simulink model "CCMHfaMotor"  (R2022b)
settings: Solver=FixedStepDiscrete  StartTime=0.0  StopTime=10.0  FixedStep=0.01  RelTol=1e-3
model workspace (MAT data in the model file):
  HfaWakeUpFlag : Simulink.Signal (value not decoded)
  NvMBlockHfaMtrCalData : Simulink.Signal (value not decoded)
  NvMBlockHfaMtrCalDataInit : Simulink.Parameter (value not decoded)
symbols that are objects in the model workspace (value not decoded): NvMBlockHfaMtrCalDataInit
undefined symbols (not defined in the file or in its folder): ComChlType, DioChannelType, HFAChlType, LinFrameType, NetworkChlType, NvMBlockId, P_DioLevelType, P_LinIfSchHandleType, P_Std_ReturnType, PowerChlType
(part 3 of 4)

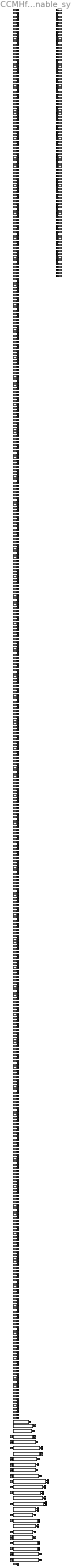
== system CCMHfaMotor/HfaMotor_Runnable_sys ==
  AcRfrPrgSafety_AcRfrPrgSafety_read : Inport
  ActRfrIdeGroup_ActRfrIdeGroup_read : Inport  Port=2
  ActRfrIdeItem_ActRfrIdeItem_read : Inport  Port=3
  ActRfrIdePN_ActRfrIdePN_read : Inport  Port=4
  ActRfrIdeSupplier_ActRfrIdeSupplier_read : Inport  Port=5
  ActRfrIdeVers_ActRfrIdeVers_read : Inport  Port=6
  ActRfrPrgDiret_ActRfrPrgDiret_read : Inport  Port=7
  ActRfrPrgGroup_ActRfrPrgGroup_read : Inport  Port=8
  ActRfrPrgItem_ActRfrPrgItem_read : Inport  Port=9
  ActRfrPrgPrgGroup_ActRfrPrgPrgGroup_read : Inport  Port=10
  ActRfrPrgPrgItem_ActRfrPrgPrgItem_read : Inport  Port=11
  ActRfrPrgRef_ActRfrPrgRef_read : Inport  Port=12
  ActRfrPrgSafetyEnable_ActRfrPrgSafetyEnable_read : Inport  Port=13
  ActRfrStaCPos_ActRfrStaCPos_read : Inport  Port=14
  ActRfrStaEmgyRun_ActRfrStaEmgyRun_read : Inport  Port=15
  ActRfrStaErr_ActRfrStaErr_read : Inport  Port=16
  ActRfrStaGroup_ActRfrStaGroup_read : Inport  Port=17
  ActRfrStaItem_ActRfrStaItem_read : Inport  Port=18
  ActRfrStaMod_ActRfrStaMod_read : Inport  Port=19
  ActRfrStaReset_ActRfrStaReset_read : Inport  Port=20
  ActRfrStaStd_ActRfrStaStd_read : Inport  Port=21
  BatSysBtrMSts_BatSysBtrMSts_read : Inport  Port=22
  HfaCcmSlaveRespB0Lin4_HfaCcmSlaveRespB0Lin4_read : Inport  Port=23
  HfaCcmSlaveRespB1Lin4_HfaCcmSlaveRespB1Lin4_read : Inport  Port=24
  HfaCcmSlaveRespB2Lin4_HfaCcmSlaveRespB2Lin4_read : Inport  Port=25
  HfaCcmSlaveRespB3Lin4_HfaCcmSlaveRespB3Lin4_read : Inport  Port=26
  HfaCcmSlaveRespB4Lin4_HfaCcmSlaveRespB4Lin4_read : Inport  Port=27
  HfaCcmSlaveRespB5Lin4_HfaCcmSlaveRespB5Lin4_read : Inport  Port=28
  HfaCcmSlaveRespB6Lin4_HfaCcmSlaveRespB6Lin4_read : Inport  Port=29
  HfaCcmSlaveRespB7Lin4_HfaCcmSlaveRespB7Lin4_read : Inport  Port=30
  HmiElecAirSwngReq_HmiElecAirSwngReq_read : Inport  Port=31
  HvacCfgForClimaCtrl_HvacCfgForHvacFrontBlowerType_read : Inport  Port=32
  HvacCfgForClimaCtrl_HvacCfgForHvacMiscExistSts_read : Inport  Port=33
  HvacCfgForClimaCtrl_HvacCfgForHvacMotorExistSts_read : Inport  Port=34
  HvacCfgForClimaCtrl_HvacCfgForHvacSensorExistSts_read : Inport  Port=35
  SbcPwrOn_SbcPwrOn_read : Inport  Port=36
  SbcWakeupSta_SbcWakeupSta_read : Inport  Port=37
  SysServCarCfgSts_SysServCarID_read : Inport  Port=38
  SysServCarCfgSts_SysServCCPAQSSts_read : Inport  Port=39
  SysServCarCfgSts_SysServCCPHEXSensorSts_read : Inport  Port=40
  SysServCarCfgSts_SysServCCPLin2AFUSts_read : Inport  Port=41
  SysServCarCfgSts_SysServCCPLin2AIUSts_read : Inport  Port=42
  SysServCarCfgSts_SysServCCPLin2CDSSts_read : Inport  Port=43
  SysServCarCfgSts_SysServCCPLin2PMSISts_read : Inport  Port=44
  SysServCarCfgSts_SysServCCPLin3VGASts_read : Inport  Port=45
  SysServCarCfgSts_SysServDUAirVentSnrSts_read : Inport  Port=46
  SysServCarCfgSts_SysServDUBlowerPwmSignSts_read : Inport  Port=47
  SysServCarCfgSts_SysServDUBlowerPwmSts_read : Inport  Port=48
  SysServCarCfgSts_SysServDUDCDefrostSts_read : Inport  Port=49
  SysServCarCfgSts_SysServDUDCModeSts_read : Inport  Port=50
  SysServCarCfgSts_SysServDUDCRecSts_read : Inport  Port=51
  SysServCarCfgSts_SysServDUDCSModeSts_read : Inport  Port=52
  SysServCarCfgSts_SysServDUDCSTLSts_read : Inport  Port=53
  SysServCarCfgSts_SysServDUDCTLSts_read : Inport  Port=54
  SysServCarCfgSts_SysServDUDCTRSts_read : Inport  Port=55
  SysServCarCfgSts_SysServDUFLFootSts_read : Inport  Port=56
  SysServCarCfgSts_SysServDUFLVentSts_read : Inport  Port=57
  SysServCarCfgSts_SysServDUFRFootSts_read : Inport  Port=58
  SysServCarCfgSts_SysServDUFRVentSts_read : Inport  Port=59
  SysServCarCfgSts_SysServDUIncarMotorSts_read : Inport  Port=60
  SysServCarCfgSts_SysServDULin1HBMFSts_read : Inport  Port=61
  SysServCarCfgSts_SysServDULin4HFAFSts_read : Inport  Port=62
  SysServCarCfgSts_SysServDUSFLFootSts_read : Inport  Port=63
  SysServCarCfgSts_SysServDUSFLVentSts_read : Inport  Port=64
  SysServCarCfgSts_SysServDUSFRFootSts_read : Inport  Port=65
  SysServCarCfgSts_SysServDUSFRVentSts_read : Inport  Port=66
  SysServCarCfgSts_SysServDUSnsrForFCmptmtTSts_read : Inport  Port=67
  SysServCarCfgSts_SysServDUSnsrForRair_read : Inport  Port=68
  SysServCarCfgSts_SysServDUSnsrForRCmptmtTSts_read : Inport  Port=69
  BatLoadBtrMSts_BatLoadBtrMSts_read : Inport  Port=70
  HfaMotSetPowerOnInit_PowerOnInit_read : Inport  Port=71
  VehModMngtGlbSafe1_VehModMngtGlbSafe1_read : Inport  Port=72
  HfaActr01ActPson_HfaActr01ActPson_read : Inport  Port=73
  HfaActr01ActPson_HfaActr01ActPson_IsUpdated_read : Inport  Port=74
  HfaActr01BlckInd_HfaActr01BlckInd_read : Inport  Port=75
  HfaActr01BlckSts_HfaActr01BlckSts_read : Inport  Port=76
  HfaActr01BusAdr_HfaActr01BusAdr_read : Inport  Port=77
  HfaActr01CoilSts_HfaActr01CoilSts_read : Inport  Port=78
  HfaActr01DrvDir_HfaActr01DrvDir_read : Inport  Port=79
  HfaActr01ElecErr_HfaActr01ElecErr_read : Inport  Port=80
  HfaActr01Mode_HfaActr01Mode_read : Inport  Port=81
  HfaActr01MovDir_HfaActr01MovDir_read : Inport  Port=82
  HfaActr01OvrTempErr_HfaActr01OvrTempErr_read : Inport  Port=83
  HfaActr01PsonSts_HfaActr01PsonSts_read : Inport  Port=84
  HfaActr01ResetSts_HfaActr01ResetSts_read : Inport  Port=85
  HfaActr01SpclFct_HfaActr01SpclFct_read : Inport  Port=86
  HfaActr01SpdSts_HfaActr01SpdSts_read : Inport  Port=87
  HfaActr01TrqSts_HfaActr01TrqSts_read : Inport  Port=88
  HfaActr01UrgcExist_HfaActr01UrgcExist_read : Inport  Port=89
  HfaActr01UrgcPson_HfaActr01UrgcPson_read : Inport  Port=90
  HfaActr01UrgcSts_HfaActr01UrgcSts_read : Inport  Port=91
  HfaActr01VoltErr_HfaActr01VoltErr_read : Inport  Port=92
  HfaActr02ActPson_HfaActr02ActPson_read : Inport  Port=93
  HfaActr02ActPson_HfaActr02ActPson_IsUpdated_read : Inport  Port=94
  HfaActr02BlckInd_HfaActr02BlckInd_read : Inport  Port=95
  HfaActr02BlckSts_HfaActr02BlckSts_read : Inport  Port=96
  HfaActr02BusAdr_HfaActr02BusAdr_read : Inport  Port=97
  HfaActr02CoilSts_HfaActr02CoilSts_read : Inport  Port=98
  HfaActr02DrvDir_HfaActr02DrvDir_read : Inport  Port=99
  HfaActr02ElecErr_HfaActr02ElecErr_read : Inport  Port=100
  HfaActr02Mode_HfaActr02Mode_read : Inport  Port=101
  HfaActr02MovDir_HfaActr02MovDir_read : Inport  Port=102
  HfaActr02OvrTempErr_HfaActr02OvrTempErr_read : Inport  Port=103
  HfaActr02PsonSts_HfaActr02PsonSts_read : Inport  Port=104
  HfaActr02ResetSts_HfaActr02ResetSts_read : Inport  Port=105
  HfaActr02SpclFct_HfaActr02SpclFct_read : Inport  Port=106
  HfaActr02SpdSts_HfaActr02SpdSts_read : Inport  Port=107
  HfaActr02TrqSts_HfaActr02TrqSts_read : Inport  Port=108
  HfaActr02UrgcExist_HfaActr02UrgcExist_read : Inport  Port=109
  HfaActr02UrgcPson_HfaActr02UrgcPson_read : Inport  Port=110
  HfaActr02UrgcSts_HfaActr02UrgcSts_read : Inport  Port=111
  HfaActr02VoltErr_HfaActr02VoltErr_read : Inport  Port=112
  HfaActr03ActPson_HfaActr03ActPson_read : Inport  Port=113
  HfaActr03ActPson_HfaActr03ActPson_IsUpdated_read : Inport  Port=114
  HfaActr03BlckInd_HfaActr03BlckInd_read : Inport  Port=115
  HfaActr03BlckSts_HfaActr03BlckSts_read : Inport  Port=116
  HfaActr03BusAdr_HfaActr03BusAdr_read : Inport  Port=117
  HfaActr03CoilSts_HfaActr03CoilSts_read : Inport  Port=118
  HfaActr03DrvDir_HfaActr03DrvDir_read : Inport  Port=119
  HfaActr03ElecErr_HfaActr03ElecErr_read : Inport  Port=120
  HfaActr03Mode_HfaActr03Mode_read : Inport  Port=121
  HfaActr03MovDir_HfaActr03MovDir_read : Inport  Port=122
  HfaActr03OvrTempErr_HfaActr03OvrTempErr_read : Inport  Port=123
  HfaActr03PsonSts_HfaActr03PsonSts_read : Inport  Port=124
  HfaActr03ResetSts_HfaActr03ResetSts_read : Inport  Port=125
  HfaActr03SpclFct_HfaActr03SpclFct_read : Inport  Port=126
  HfaActr03SpdSts_HfaActr03SpdSts_read : Inport  Port=127
  HfaActr03TrqSts_HfaActr03TrqSts_read : Inport  Port=128
  HfaActr03UrgcExist_HfaActr03UrgcExist_read : Inport  Port=129
  HfaActr03UrgcPson_HfaActr03UrgcPson_read : Inport  Port=130
  HfaActr03UrgcSts_HfaActr03UrgcSts_read : Inport  Port=131
  HfaActr03VoltErr_HfaActr03VoltErr_read : Inport  Port=132
  HfaActr04ActPson_HfaActr04ActPson_read : Inport  Port=133
  HfaActr04ActPson_HfaActr04ActPson_IsUpdated_read : Inport  Port=134
  HfaActr04BlckInd_HfaActr04BlckInd_read : Inport  Port=135
  HfaActr04BlckSts_HfaActr04BlckSts_read : Inport  Port=136
  HfaActr04BusAdr_HfaActr04BusAdr_read : Inport  Port=137
  HfaActr04CoilSts_HfaActr04CoilSts_read : Inport  Port=138
  HfaActr04DrvDir_HfaActr04DrvDir_read : Inport  Port=139
  HfaActr04ElecErr_HfaActr04ElecErr_read : Inport  Port=140
  HfaActr04Mode_HfaActr04Mode_read : Inport  Port=141
  HfaActr04MovDir_HfaActr04MovDir_read : Inport  Port=142
  HfaActr04OvrTempErr_HfaActr04OvrTempErr_read : Inport  Port=143
  HfaActr04PsonSts_HfaActr04PsonSts_read : Inport  Port=144
  HfaActr04ResetSts_HfaActr04ResetSts_read : Inport  Port=145
  HfaActr04SpclFct_HfaActr04SpclFct_read : Inport  Port=146
  HfaActr04SpdSts_HfaActr04SpdSts_read : Inport  Port=147
  HfaActr04TrqSts_HfaActr04TrqSts_read : Inport  Port=148
  HfaActr04UrgcExist_HfaActr04UrgcExist_read : Inport  Port=149
  HfaActr04UrgcPson_HfaActr04UrgcPson_read : Inport  Port=150
  HfaActr04UrgcSts_HfaActr04UrgcSts_read : Inport  Port=151
  HfaActr04VoltErr_HfaActr04VoltErr_read : Inport  Port=152
  HfaActr05ActPson_HfaActr05ActPson_read : Inport  Port=153
  HfaActr05ActPson_HfaActr05ActPson_IsUpdated_read : Inport  Port=154
  HfaActr05BlckInd_HfaActr05BlckInd_read : Inport  Port=155
  HfaActr05BlckSts_HfaActr05BlckSts_read : Inport  Port=156
  HfaActr05BusAdr_HfaActr05BusAdr_read : Inport  Port=157
  HfaActr05CoilSts_HfaActr05CoilSts_read : Inport  Port=158
  HfaActr05DrvDir_HfaActr05DrvDir_read : Inport  Port=159
  HfaActr05ElecErr_HfaActr05ElecErr_read : Inport  Port=160
  HfaActr05Mode_HfaActr05Mode_read : Inport  Port=161
  HfaActr05MovDir_HfaActr05MovDir_read : Inport  Port=162
  HfaActr05OvrTempErr_HfaActr05OvrTempErr_read : Inport  Port=163
  HfaActr05PsonSts_HfaActr05PsonSts_read : Inport  Port=164
  HfaActr05ResetSts_HfaActr05ResetSts_read : Inport  Port=165
  HfaActr05SpclFct_HfaActr05SpclFct_read : Inport  Port=166
  HfaActr05SpdSts_HfaActr05SpdSts_read : Inport  Port=167
  HfaActr05TrqSts_HfaActr05TrqSts_read : Inport  Port=168
  HfaActr05UrgcExist_HfaActr05UrgcExist_read : Inport  Port=169
  HfaActr05UrgcPson_HfaActr05UrgcPson_read : Inport  Port=170
  HfaActr05UrgcSts_HfaActr05UrgcSts_read : Inport  Port=171
  HfaActr05VoltErr_HfaActr05VoltErr_read : Inport  Port=172
  HfaActr06ActPson_HfaActr06ActPson_read : Inport  Port=173
  HfaActr06ActPson_HfaActr06ActPson_IsUpdated_read : Inport  Port=174
  HfaActr06BlckInd_HfaActr06BlckInd_read : Inport  Port=175
  HfaActr06BlckSts_HfaActr06BlckSts_read : Inport  Port=176
  HfaActr06BusAdr_HfaActr06BusAdr_read : Inport  Port=177
  HfaActr06CoilSts_HfaActr06CoilSts_read : Inport  Port=178
  HfaActr06DrvDir_HfaActr06DrvDir_read : Inport  Port=179
  HfaActr06ElecErr_HfaActr06ElecErr_read : Inport  Port=180
  HfaActr06Mode_HfaActr06Mode_read : Inport  Port=181
  HfaActr06MovDir_HfaActr06MovDir_read : Inport  Port=182
  HfaActr06OvrTempErr_HfaActr06OvrTempErr_read : Inport  Port=183
  HfaActr06PsonSts_HfaActr06PsonSts_read : Inport  Port=184
  HfaActr06ResetSts_HfaActr06ResetSts_read : Inport  Port=185
  HfaActr06SpclFct_HfaActr06SpclFct_read : Inport  Port=186
  HfaActr06SpdSts_HfaActr06SpdSts_read : Inport  Port=187
  HfaActr06TrqSts_HfaActr06TrqSts_read : Inport  Port=188
  HfaActr06UrgcExist_HfaActr06UrgcExist_read : Inport  Port=189
  HfaActr06UrgcPson_HfaActr06UrgcPson_read : Inport  Port=190
  HfaActr06UrgcSts_HfaActr06UrgcSts_read : Inport  Port=191
  HfaActr06VoltErr_HfaActr06VoltErr_read : Inport  Port=192
  HfaActr07ActPson_HfaActr07ActPson_read : Inport  Port=193
  HfaActr07ActPson_HfaActr07ActPson_IsUpdated_read : Inport  Port=194
  HfaActr07BlckInd_HfaActr07BlckInd_read : Inport  Port=195
  HfaActr07BlckSts_HfaActr07BlckSts_read : Inport  Port=196
  HfaActr07BusAdr_HfaActr07BusAdr_read : Inport  Port=197
  HfaActr07CoilSts_HfaActr07CoilSts_read : Inport  Port=198
  HfaActr07DrvDir_HfaActr07DrvDir_read : Inport  Port=199
  HfaActr07ElecErr_HfaActr07ElecErr_read : Inport  Port=200
  HfaActr07Mode_HfaActr07Mode_read : Inport  Port=201
  HfaActr07MovDir_HfaActr07MovDir_read : Inport  Port=202
  HfaActr07OvrTempErr_HfaActr07OvrTempErr_read : Inport  Port=203
  HfaActr07PsonSts_HfaActr07PsonSts_read : Inport  Port=204
  HfaActr07ResetSts_HfaActr07ResetSts_read : Inport  Port=205
  HfaActr07SpclFct_HfaActr07SpclFct_read : Inport  Port=206
  HfaActr07SpdSts_HfaActr07SpdSts_read : Inport  Port=207
  HfaActr07TrqSts_HfaActr07TrqSts_read : Inport  Port=208
  HfaActr07UrgcExist_HfaActr07UrgcExist_read : Inport  Port=209
  HfaActr07UrgcPson_HfaActr07UrgcPson_read : Inport  Port=210
  HfaActr07UrgcSts_HfaActr07UrgcSts_read : Inport  Port=211
  HfaActr07VoltErr_HfaActr07VoltErr_read : Inport  Port=212
  HfaActr08ActPson_HfaActr08ActPson_read : Inport  Port=213
  HfaActr08ActPson_HfaActr08ActPson_IsUpdated_read : Inport  Port=214
  HfaActr08BlckInd_HfaActr08BlckInd_read : Inport  Port=215
  HfaActr08BlckSts_HfaActr08BlckSts_read : Inport  Port=216
  HfaActr08BusAdr_HfaActr08BusAdr_read : Inport  Port=217
  HfaActr08CoilSts_HfaActr08CoilSts_read : Inport  Port=218
  HfaActr08DrvDir_HfaActr08DrvDir_read : Inport  Port=219
  HfaActr08ElecErr_HfaActr08ElecErr_read : Inport  Port=220
  HfaActr08Mode_HfaActr08Mode_read : Inport  Port=221
  HfaActr08MovDir_HfaActr08MovDir_read : Inport  Port=222
  HfaActr08OvrTempErr_HfaActr08OvrTempErr_read : Inport  Port=223
  HfaActr08PsonSts_HfaActr08PsonSts_read : Inport  Port=224
  HfaActr08ResetSts_HfaActr08ResetSts_read : Inport  Port=225
  HfaActr08SpclFct_HfaActr08SpclFct_read : Inport  Port=226
  HfaActr08SpdSts_HfaActr08SpdSts_read : Inport  Port=227
  HfaActr08TrqSts_HfaActr08TrqSts_read : Inport  Port=228
  HfaActr08UrgcExist_HfaActr08UrgcExist_read : Inport  Port=229
  HfaActr08UrgcPson_HfaActr08UrgcPson_read : Inport  Port=230
  HfaActr08UrgcSts_HfaActr08UrgcSts_read : Inport  Port=231
  HfaActr08VoltErr_HfaActr08VoltErr_read : Inport  Port=232
  HfaActr09ActPson_HfaActr09ActPson_read : Inport  Port=233
  HfaActr09ActPson_HfaActr09ActPson_IsUpdated_read : Inport  Port=234
  HfaActr09BlckInd_HfaActr09BlckInd_read : Inport  Port=235
  HfaActr09BlckSts_HfaActr09BlckSts_read : Inport  Port=236
  HfaActr09BusAdr_HfaActr09BusAdr_read : Inport  Port=237
  HfaActr09CoilSts_HfaActr09CoilSts_read : Inport  Port=238
  HfaActr09DrvDir_HfaActr09DrvDir_read : Inport  Port=239
  HfaActr09ElecErr_HfaActr09ElecErr_read : Inport  Port=240
  HfaActr09Mode_HfaActr09Mode_read : Inport  Port=241
  HfaActr09MovDir_HfaActr09MovDir_read : Inport  Port=242
  HfaActr09OvrTempErr_HfaActr09OvrTempErr_read : Inport  Port=243
  HfaActr09PsonSts_HfaActr09PsonSts_read : Inport  Port=244
  HfaActr09ResetSts_HfaActr09ResetSts_read : Inport  Port=245
  HfaActr09SpclFct_HfaActr09SpclFct_read : Inport  Port=246
  HfaActr09SpdSts_HfaActr09SpdSts_read : Inport  Port=247
  HfaActr09TrqSts_HfaActr09TrqSts_read : Inport  Port=248
  HfaActr09UrgcExist_HfaActr09UrgcExist_read : Inport  Port=249
  HfaActr09UrgcPson_HfaActr09UrgcPson_read : Inport  Port=250
  HfaActr09UrgcSts_HfaActr09UrgcSts_read : Inport  Port=251
  HfaActr09VoltErr_HfaActr09VoltErr_read : Inport  Port=252
  HfaActr10ActPson_HfaActr10ActPson_read : Inport  Port=253
  HfaActr10ActPson_HfaActr10ActPson_IsUpdated_read : Inport  Port=254
  HfaActr10BlckInd_HfaActr10BlckInd_read : Inport  Port=255
  HfaActr10BlckSts_HfaActr10BlckSts_read : Inport  Port=256
  HfaActr10BusAdr_HfaActr10BusAdr_read : Inport  Port=257
  HfaActr10CoilSts_HfaActr10CoilSts_read : Inport  Port=258
  HfaActr10DrvDir_HfaActr10DrvDir_read : Inport  Port=259
  HfaActr10ElecErr_HfaActr10ElecErr_read : Inport  Port=260
  HfaActr10Mode_HfaActr10Mode_read : Inport  Port=261
  HfaActr10MovDir_HfaActr10MovDir_read : Inport  Port=262
  HfaActr10OvrTempErr_HfaActr10OvrTempErr_read : Inport  Port=263
  HfaActr10PsonSts_HfaActr10PsonSts_read : Inport  Port=264
  HfaActr10ResetSts_HfaActr10ResetSts_read : Inport  Port=265
  HfaActr10SpclFct_HfaActr10SpclFct_read : Inport  Port=266
  HfaActr10SpdSts_HfaActr10SpdSts_read : Inport  Port=267
  HfaActr10TrqSts_HfaActr10TrqSts_read : Inport  Port=268
  HfaActr10UrgcExist_HfaActr10UrgcExist_read : Inport  Port=269
  HfaActr10UrgcPson_HfaActr10UrgcPson_read : Inport  Port=270
  HfaActr10UrgcSts_HfaActr10UrgcSts_read : Inport  Port=271
  HfaActr10VoltErr_HfaActr10VoltErr_read : Inport  Port=272
  HfaActr11ActPson_HfaActr11ActPson_read : Inport  Port=273
  HfaActr11ActPson_HfaActr11ActPson_IsUpdated_read : Inport  Port=274
  HfaActr11BlckInd_HfaActr11BlckInd_read : Inport  Port=275
  HfaActr11BlckSts_HfaActr11BlckSts_read : Inport  Port=276
  HfaActr11BusAdr_HfaActr11BusAdr_read : Inport  Port=277
  HfaActr11CoilSts_HfaActr11CoilSts_read : Inport  Port=278
  HfaActr11DrvDir_HfaActr11DrvDir_read : Inport  Port=279
  HfaActr11ElecErr_HfaActr11ElecErr_read : Inport  Port=280
  HfaActr11Mode_HfaActr11Mode_read : Inport  Port=281
  HfaActr11MovDir_HfaActr11MovDir_read : Inport  Port=282
  HfaActr11OvrTempErr_HfaActr11OvrTempErr_read : Inport  Port=283
  HfaActr11PsonSts_HfaActr11PsonSts_read : Inport  Port=284
  HfaActr11ResetSts_HfaActr11ResetSts_read : Inport  Port=285
  HfaActr11SpclFct_HfaActr11SpclFct_read : Inport  Port=286
  HfaActr11SpdSts_HfaActr11SpdSts_read : Inport  Port=287
  HfaActr11TrqSts_HfaActr11TrqSts_read : Inport  Port=288
  HfaActr11UrgcExist_HfaActr11UrgcExist_read : Inport  Port=289
  HfaActr11UrgcPson_HfaActr11UrgcPson_read : Inport  Port=290
  HfaActr11UrgcSts_HfaActr11UrgcSts_read : Inport  Port=291
  HfaActr11VoltErr_HfaActr11VoltErr_read : Inport  Port=292
  HfaActr12ActPson_HfaActr12ActPson_read : Inport  Port=293
  HfaActr12ActPson_HfaActr12ActPson_IsUpdated_read : Inport  Port=294
  HfaActr12BlckInd_HfaActr12BlckInd_read : Inport  Port=295
  HfaActr12BlckSts_HfaActr12BlckSts_read : Inport  Port=296
  HfaActr12BusAdr_HfaActr12BusAdr_read : Inport  Port=297
  HfaActr12CoilSts_HfaActr12CoilSts_read : Inport  Port=298
  HfaActr12DrvDir_HfaActr12DrvDir_read : Inport  Port=299
  HfaActr12ElecErr_HfaActr12ElecErr_read : Inport  Port=300
  HfaActr12Mode_HfaActr12Mode_read : Inport  Port=301
  HfaActr12MovDir_HfaActr12MovDir_read : Inport  Port=302
  HfaActr12OvrTempErr_HfaActr12OvrTempErr_read : Inport  Port=303
  HfaActr12PsonSts_HfaActr12PsonSts_read : Inport  Port=304
  HfaActr12ResetSts_HfaActr12ResetSts_read : Inport  Port=305
  HfaActr12SpclFct_HfaActr12SpclFct_read : Inport  Port=306
  HfaActr12SpdSts_HfaActr12SpdSts_read : Inport  Port=307
  HfaActr12TrqSts_HfaActr12TrqSts_read : Inport  Port=308
  HfaActr12UrgcExist_HfaActr12UrgcExist_read : Inport  Port=309
  HfaActr12UrgcPson_HfaActr12UrgcPson_read : Inport  Port=310
  HfaActr12UrgcSts_HfaActr12UrgcSts_read : Inport  Port=311
  HfaActr12VoltErr_HfaActr12VoltErr_read : Inport  Port=312
  HfaActr13ActPson_HfaActr13ActPson_read : Inport  Port=313
  HfaActr13ActPson_HfaActr13ActPson_IsUpdated_read : Inport  Port=314
  HfaActr13BlckInd_HfaActr13BlckInd_read : Inport  Port=315
  HfaActr13BlckSts_HfaActr13BlckSts_read : Inport  Port=316
  HfaActr13BusAdr_HfaActr13BusAdr_read : Inport  Port=317
  HfaActr13CoilSts_HfaActr13CoilSts_read : Inport  Port=318
  HfaActr13DrvDir_HfaActr13DrvDir_read : Inport  Port=319
  HfaActr13ElecErr_HfaActr13ElecErr_read : Inport  Port=320
  HfaActr13Mode_HfaActr13Mode_read : Inport  Port=321
  HfaActr13MovDir_HfaActr13MovDir_read : Inport  Port=322
  HfaActr13OvrTempErr_HfaActr13OvrTempErr_read : Inport  Port=323
  HfaActr13PsonSts_HfaActr13PsonSts_read : Inport  Port=324
  HfaActr13ResetSts_HfaActr13ResetSts_read : Inport  Port=325
  HfaActr13SpclFct_HfaActr13SpclFct_read : Inport  Port=326
  HfaActr13SpdSts_HfaActr13SpdSts_read : Inport  Port=327
  HfaActr13TrqSts_HfaActr13TrqSts_read : Inport  Port=328
  HfaActr13UrgcExist_HfaActr13UrgcExist_read : Inport  Port=329
  HfaActr13UrgcPson_HfaActr13UrgcPson_read : Inport  Port=330
  HfaActr13UrgcSts_HfaActr13UrgcSts_read : Inport  Port=331
  HfaActr13VoltErr_HfaActr13VoltErr_read : Inport  Port=332
  HfaActr14ActPson_HfaActr14ActPson_read : Inport  Port=333
  HfaActr14ActPson_HfaActr14ActPson_IsUpdated_read : Inport  Port=334
  HfaActr14BlckInd_HfaActr14BlckInd_read : Inport  Port=335
  HfaActr14BlckSts_HfaActr14BlckSts_read : Inport  Port=336
  HfaActr14BusAdr_HfaActr14BusAdr_read : Inport  Port=337
  HfaActr14CoilSts_HfaActr14CoilSts_read : Inport  Port=338
  HfaActr14DrvDir_HfaActr14DrvDir_read : Inport  Port=339
  HfaActr14ElecErr_HfaActr14ElecErr_read : Inport  Port=340
  HfaActr14Mode_HfaActr14Mode_read : Inport  Port=341
  HfaActr14MovDir_HfaActr14MovDir_read : Inport  Port=342
  HfaActr14OvrTempErr_HfaActr14OvrTempErr_read : Inport  Port=343
  HfaActr14PsonSts_HfaActr14PsonSts_read : Inport  Port=344
  HfaActr14ResetSts_HfaActr14ResetSts_read : Inport  Port=345
  HfaActr14SpclFct_HfaActr14SpclFct_read : Inport  Port=346
  HfaActr14SpdSts_HfaActr14SpdSts_read : Inport  Port=347
  HfaActr14TrqSts_HfaActr14TrqSts_read : Inport  Port=348
  HfaActr14UrgcExist_HfaActr14UrgcExist_read : Inport  Port=349
  HfaActr14UrgcPson_HfaActr14UrgcPson_read : Inport  Port=350
  HfaActr14UrgcSts_HfaActr14UrgcSts_read : Inport  Port=351
  HfaActr14VoltErr_HfaActr14VoltErr_read : Inport  Port=352
  HfaActr15ActPson_HfaActr15ActPson_read : Inport  Port=353
  HfaActr15ActPson_HfaActr15ActPson_IsUpdated_read : Inport  Port=354
  HfaActr15BlckInd_HfaActr15BlckInd_read : Inport  Port=355
  HfaActr15BlckSts_HfaActr15BlckSts_read : Inport  Port=356
  HfaActr15BusAdr_HfaActr15BusAdr_read : Inport  Port=357
  HfaActr15CoilSts_HfaActr15CoilSts_read : Inport  Port=358
  HfaActr15DrvDir_HfaActr15DrvDir_read : Inport  Port=359
  HfaActr15ElecErr_HfaActr15ElecErr_read : Inport  Port=360
  HfaActr15Mode_HfaActr15Mode_read : Inport  Port=361
  HfaActr15MovDir_HfaActr15MovDir_read : Inport  Port=362
  HfaActr15OvrTempErr_HfaActr15OvrTempErr_read : Inport  Port=363
  HfaActr15PsonSts_HfaActr15PsonSts_read : Inport  Port=364
  HfaActr15ResetSts_HfaActr15ResetSts_read : Inport  Port=365
  HfaActr15SpclFct_HfaActr15SpclFct_read : Inport  Port=366
  HfaActr15SpdSts_HfaActr15SpdSts_read : Inport  Port=367
  HfaActr15TrqSts_HfaActr15TrqSts_read : Inport  Port=368
  HfaActr15UrgcExist_HfaActr15UrgcExist_read : Inport  Port=369
  HfaActr15UrgcPson_HfaActr15UrgcPson_read : Inport  Port=370
  HfaActr15UrgcSts_HfaActr15UrgcSts_read : Inport  Port=371
  HfaActr15VoltErr_HfaActr15VoltErr_read : Inport  Port=372
  HvacCfgForClimaCtrl_HvacCfgForAQSTypes_read : Inport  Port=373
  HvacCfgForClimaCtrl_HvacCfgForHvacLowSideRETXVESts_read : Inport  Port=374
  HvacCfgForClimaCtrl_HvacCfgForHvacReBlowerType_read : Inport  Port=375
  HvacCfgForClimaCtrl_HvacCfgForHvacReVentPstn_read : Inport  Port=376
  HvacCfgForClimaCtrl_HvacCfgForHvacSnsrRCmptmtESts_read : Inport  Port=377
  HvacCfgForClimaCtrl_HvacCfgForHvacSnsrReHexESts_read : Inport  Port=378
  Lin1ActvReady_Lin1ActvReady_read : Inport  Port=379
  Lin2ActvReady_Lin2ActvReady_read : Inport  Port=380
  ReHvacActrActPson_ReHvacActrActPson_read : Inport  Port=381
  ReHvacActrActPson_ReHvacActrActPson_IsUpdated_read : Inport  Port=382
  ReHvacActrBlckInd_ReHvacActrBlckInd_read : Inport  Port=383
  ReHvacActrBlckSts_ReHvacActrBlckSts_read : Inport  Port=384
  ReHvacActrBusAdr_ReHvacActrBusAdr_read : Inport  Port=385
  ReHvacActrCoilSts_ReHvacActrCoilSts_read : Inport  Port=386
  ReHvacActrDrvDir_ReHvacActrDrvDir_read : Inport  Port=387
  ReHvacActrElecErr_ReHvacActrElecErr_read : Inport  Port=388
  ReHvacActrMode_ReHvacActrMode_read : Inport  Port=389
  ReHvacActrMovDir_ReHvacActrMovDir_read : Inport  Port=390
  ReHvacActrOvrTempErr_ReHvacActrOvrTempErr_read : Inport  Port=391
  ReHvacActrPsonSts_ReHvacActrPsonSts_read : Inport  Port=392
  ReHvacActrResetSts_ReHvacActrResetSts_read : Inport  Port=393
  ReHvacActrSpclFct_ReHvacActrSpclFct_read : Inport  Port=394
  ReHvacActrSpdSts_ReHvacActrSpdSts_read : Inport  Port=395
  ReHvacActrTrqSts_ReHvacActrTrqSts_read : Inport  Port=396
  ReHvacActrUrgcExist_ReHvacActrUrgcExist_read : Inport  Port=397
  ReHvacActrUrgcPson_ReHvacActrUrgcPson_read : Inport  Port=398
  ReHvacActrUrgcSts_ReHvacActrUrgcSts_read : Inport  Port=399
  ReHvacActrVoltErr_ReHvacActrVoltErr_read : Inport  Port=400
  SysServCarCfgSts_SysServCCPBGMNum_read : Inport  Port=401
  SysServCarCfgSts_SysServCCPLin2AQSSts_read : Inport  Port=402
  SysServCarCfgSts_SysServDUBlowerPwmSignTypeSts_read : Inport  Port=403
  SysServCarCfgSts_SysServDUDCFLMdeSts_read : Inport  Port=404
  SysServCarCfgSts_SysServDULin4HFAFStsH_read : Inport  Port=405
  SysServCarCfgSts_SysServDUReBlowerPwmSignSts_read : Inport  Port=406
  SysServCarCfgSts_SysServDUReBlowerPwmSts_read : Inport  Port=407
  SysServCarCfgSts_SysServFloatingMotorSts_read : Inport  Port=408
  SysServCarCfgSts_SysServFrntHEXSnsrPosn_read : Inport  Port=409
  SysServCarCfgSts_SysServLowSideRETXVSts_read : Inport  Port=410
  SysServCarCfgSts_SysServReHEXSnsrPosn_read : Inport  Port=411
  SysServCarCfgSts_SysServRHFASts_read : Inport  Port=412
  SysServCarCfgSts_SysServSnsrForFCmptmtTType_read : Inport  Port=413
  WtrActrLeActPson_WtrActrLeActPson_read : Inport  Port=414
  WtrActrLeBlckInd_WtrActrLeBlckInd_read : Inport  Port=415
  WtrActrLeBlckSts_WtrActrLeBlckSts_read : Inport  Port=416
  WtrActrLeBusAdr_WtrActrLeBusAdr_read : Inport  Port=417
  WtrActrLeCoilSts_WtrActrLeCoilSts_read : Inport  Port=418
  WtrActrLeDir_WtrActrLeDir_read : Inport  Port=419
  WtrActrLeElecErr_WtrActrLeElecErr_read : Inport  Port=420
  WtrActrLeMode_WtrActrLeMode_read : Inport  Port=421
  WtrActrLeMovDir_WtrActrLeMovDir_read : Inport  Port=422
  WtrActrLeOvrTempErr_WtrActrLeOvrTempErr_read : Inport  Port=423
  WtrActrLePsonSts_WtrActrLePsonSts_read : Inport  Port=424
  WtrActrLeResetSts_WtrActrLeResetSts_read : Inport  Port=425
  WtrActrLeSpclFct_WtrActrLeSpclFct_read : Inport  Port=426
  WtrActrLeSpdSts_WtrActrLeSpdSts_read : Inport  Port=427
  WtrActrLeTrqSts_WtrActrLeTrqSts_read : Inport  Port=428
  WtrActrLeUrgcExist_WtrActrLeUrgcExist_read : Inport  Port=429
  WtrActrLeUrgcPson_WtrActrLeUrgcPson_read : Inport  Port=430
  WtrActrLeUrgcSts_WtrActrLeUrgcSts_read : Inport  Port=431
  WtrActrLeVoltErr_WtrActrLeVoltErr_read : Inport  Port=432
  WtrActrRiActPson_WtrActrRiActPson_read : Inport  Port=433
  WtrActrRiBlckInd_WtrActrRiBlckInd_read : Inport  Port=434
  WtrActrRiBlckSts_WtrActrRiBlckSts_read : Inport  Port=435
  WtrActrRiBusAdr_WtrActrRiBusAdr_read : Inport  Port=436
  WtrActrRiCoilSts_WtrActrRiCoilSts_read : Inport  Port=437
  WtrActrRiDir_WtrActrRiDir_read : Inport  Port=438
  WtrActrRiElecErr_WtrActrRiElecErr_read : Inport  Port=439
  WtrActrRiMode_WtrActrRiMode_read : Inport  Port=440
  WtrActrRiMovDir_WtrActrRiMovDir_read : Inport  Port=441
  WtrActrRiOvrTempErr_WtrActrRiOvrTempErr_read : Inport  Port=442
  WtrActrRiPsonSts_WtrActrRiPsonSts_read : Inport  Port=443
  WtrActrRiResetSts_WtrActrRiResetSts_read : Inport  Port=444
  WtrActrRiSpclFct_WtrActrRiSpclFct_read : Inport  Port=445
  WtrActrRiSpdSts_WtrActrRiSpdSts_read : Inport  Port=446
  WtrActrRiTrqSts_WtrActrRiTrqSts_read : Inport  Port=447
  WtrActrRiUrgcExist_WtrActrRiUrgcExist_read : Inport  Port=448
  WtrActrRiUrgcPson_WtrActrRiUrgcPson_read : Inport  Port=449
  WtrActrRiUrgcSts_WtrActrRiUrgcSts_read : Inport  Port=450
  WtrActrRiVoltErr_WtrActrRiVoltErr_read : Inport  Port=451
  BswLifeCycle_LifeCycle_read : Inport  Port=452
  function : TriggerPort  TriggerType=function-call  VariantControl=(inherit)  SampleTimeType=periodic  SampleTime=-1
  DioServ_PowerOffInitChannel : FunctionCaller  FunctionPrototype=ERR = DioServ_PowerOffInitChannel()  OutputArgumentSpecifications=P_Std_ReturnType
  DioServ_PowerOnInitChannel : FunctionCaller  FunctionPrototype=ERR = DioServ_PowerOnInitChannel()  OutputArgumentSpecifications=P_Std_ReturnType
  DioServ_ReadChannel : FunctionCaller  FunctionPrototype=[Level,ERR] = DioServ_ReadChannel(ChannelId)  InputArgumentSpecifications=DioChannelType(0)  OutputArgumentSpecifications=P_DioLevelType, P_Std_ReturnType
  DioServ_WriteChannel : FunctionCaller  FunctionPrototype=ERR = DioServ_WriteChannel(ChannelId,Level)  InputArgumentSpecifications=DioChannelType(0), P_DioLevelType  OutputArgumentSpecifications=P_Std_ReturnType
  Ground_{1..113} (113 alike) : Ground
  LinIfServ_GetScheduleInfo : FunctionCaller  FunctionPrototype=[ScheduleInfo,ERR] = LinIfServ_GetScheduleInfo(Channel)  InputArgumentSpecifications=NetworkChlType(0)  OutputArgumentSpecifications=P_LinIfScheduleInfoType, P_Std_ReturnType
  LinSMServ_ScheduleRequest : FunctionCaller  FunctionPrototype=ERR = LinSMServ_ScheduleRequest(network,schedule)  InputArgumentSpecifications=NetworkChlType(0), P_LinIfSchHandleType  OutputArgumentSpecifications=P_Std_ReturnType
  LinService_GetLin2WakeupStatus : FunctionCaller  FunctionPrototype=[WakSta,ERR] = LinService_GetLin2WakeupStatus()  OutputArgumentSpecifications=uint8(1), P_Std_ReturnType
  LinService_SendLin3dFrame : FunctionCaller  FunctionPrototype=ERR = LinService_SendLin3dFrame(channel,data)  InputArgumentSpecifications=ComChlType(0), uint8([1])  OutputArgumentSpecifications=P_Std_ReturnType
  LinService_SetLin3cFrame : FunctionCaller  FunctionPrototype=ERR = LinService_SetLin3cFrame(Channel,Data)  InputArgumentSpecifications=ComChlType(0), uint8([1])  OutputArgumentSpecifications=P_Std_ReturnType
  LinService_SetLinTable : FunctionCaller  FunctionPrototype=ERR = LinService_SetLinTable(Channel,Table)  InputArgumentSpecifications=ComChlType(0), uint8(1)  OutputArgumentSpecifications=P_Std_ReturnType
  MirrorLin4ErrServ_GetHfaCcmLin4Fr01NoResp : FunctionCaller  FunctionPrototype=[ErrFlag,ERR] = MirrorLin4ErrServ_GetHfaCcmLin4Fr01NoResp(Channel)  InputArgumentSpecifications=HFAChlType(0)  OutputArgumentSpecifications=uint8(1), P_Std_ReturnType
  MirrorLin4ErrServ_GetHfaRxErrCurSts : FunctionCaller  FunctionPrototype=[ErrFlag,ERR] = MirrorLin4ErrServ_GetHfaRxErrCurSts(Channel)  InputArgumentSpecifications=HFAChlType(0)  OutputArgumentSpecifications=uint8(1), P_Std_ReturnType
  MirrorLin4ErrServ_GetHfaRxErrSta : FunctionCaller  FunctionPrototype=[ErrFlag,ERR] = MirrorLin4ErrServ_GetHfaRxErrSta(Channel)  InputArgumentSpecifications=HFAChlType(0)  OutputArgumentSpecifications=uint8(1), P_Std_ReturnType
  MirrorLin4ErrServ_GetLinBusTxErrSta : FunctionCaller  FunctionPrototype=[ErrFlag,ERR] = MirrorLin4ErrServ_GetLinBusTxErrSta()  OutputArgumentSpecifications=uint8(1), P_Std_ReturnType
  MirrorLinFrameServ_GetRespSta : FunctionCaller  FunctionPrototype=[RxAckSts,RxData,ERR] = MirrorLinFrameServ_GetRespSta(FrameId)  InputArgumentSpecifications=LinFrameType(0)  OutputArgumentSpecifications=P_LinAckType, P_LinDataType, P_Std_ReturnType
  NvMServ_HfaMtrCalReadBlock : FunctionCaller  FunctionPrototype=[Data,ERR] = NvMServ_HfaMtrCalReadBlock()  OutputArgumentSpecifications=uint8([1]), P_Std_ReturnType
  NvMServ_HfaMtrCalWriteBlock : FunctionCaller  FunctionPrototype=ERR = NvMServ_HfaMtrCalWriteBlock(Data)  InputArgumentSpecifications=uint8([1])  OutputArgumentSpecifications=P_Std_ReturnType
  NvMServ_NvMNotifyJobWorking : FunctionCaller  FunctionPrototype=[Status,ERR] = NvMServ_NvMNotifyJobWorking(BlockId)  InputArgumentSpecifications=NvMBlockId(0)  OutputArgumentSpecifications=uint8(1), P_Std_ReturnType
  NvMServ_VgaMtrCalReadBlock : FunctionCaller  FunctionPrototype=[Data,ERR] = NvMServ_VgaMtrCalReadBlock()  OutputArgumentSpecifications=uint8([1]), P_Std_ReturnType
  NvMServ_VgaMtrCalWriteBlock : FunctionCaller  FunctionPrototype=ERR = NvMServ_VgaMtrCalWriteBlock(Data)  InputArgumentSpecifications=uint8([1])  OutputArgumentSpecifications=P_Std_ReturnType
  NvMServ_WriteBlock : FunctionCaller  FunctionPrototype=ERR = NvMServ_WriteBlock(BlockId,Data)  InputArgumentSpecifications=NvMBlockId(0), uint8([1])  OutputArgumentSpecifications=P_Std_ReturnType
  PA_NvMBlockHfaMtrCalDataInit : Constant  Value=NvMBlockHfaMtrCalDataInit
  PwrParaService_GetMotorCailCnt : FunctionCaller  FunctionPrototype=[Cnt,ERR] = PwrParaService_GetMotorCailCnt(Channel)  InputArgumentSpecifications=PowerChlType(0)  OutputArgumentSpecifications=uint8(1), P_Std_ReturnType
  PwrParaService_GetMotorCailNum : FunctionCaller  FunctionPrototype=[Num,ERR] = PwrParaService_GetMotorCailNum(Channel)  InputArgumentSpecifications=PowerChlType(0)  OutputArgumentSpecifications=uint8(1), P_Std_ReturnType
  PwrParaService_SetMotorCailCnt : FunctionCaller  FunctionPrototype=ERR = PwrParaService_SetMotorCailCnt(Channel,Cnt)  InputArgumentSpecifications=PowerChlType(0), uint8(1)  OutputArgumentSpecifications=P_Std_ReturnType
  PwrParaService_SetMotorCailNum : FunctionCaller  FunctionPrototype=ERR = PwrParaService_SetMotorCailNum(Channel,Num)  InputArgumentSpecifications=PowerChlType(0), uint8(1)  OutputArgumentSpecifications=P_Std_ReturnType
  Terminator_{1..492} (492 alike) : Terminator
  ActCfrAddrFinalAddr_ActCfrAddrFinalAddr_write : Outport
      settings: EnsureOutportIsVirtual=on
  ActCfrAddrGroup_ActCfrAddrGroup_write : Outport  Port=2
      settings: EnsureOutportIsVirtual=on
  ActCfrAddrItem_ActCfrAddrItem_write : Outport  Port=3
      settings: EnsureOutportIsVirtual=on
  ActCfrIniCPos_ActCfrIniCPos_write : Outport  Port=4
      settings: EnsureOutportIsVirtual=on
  ActCfrIniGroup_ActCfrIniGroup_write : Outport  Port=5
      settings: EnsureOutportIsVirtual=on
  ActCfrIniItem_ActCfrIniItem_write : Outport  Port=6
      settings: EnsureOutportIsVirtual=on
  ActCfrIniResvd_ActCfrIniResvd_write : Outport  Port=7
      settings: EnsureOutportIsVirtual=on
  ActCfrModGroup_ActCfrModGroup_write : Outport  Port=8
      settings: EnsureOutportIsVirtual=on
  ActCfrModItem_ActCfrModItem_write : Outport  Port=9
      settings: EnsureOutportIsVirtual=on
  ActCfrModNewMod_ActCfrModNewMod_write : Outport  Port=10
      settings: EnsureOutportIsVirtual=on
  ActCfrModResvd1_ActCfrModResvd1_write : Outport  Port=11
      settings: EnsureOutportIsVirtual=on
  ActCfrModResvd2_ActCfrModResvd2_write : Outport  Port=12
      settings: EnsureOutportIsVirtual=on
  ActCfrPrgDiret_ActCfrPrgDiret_write : Outport  Port=13
      settings: EnsureOutportIsVirtual=on
  ActCfrPrgGroup_ActCfrPrgGroup_write : Outport  Port=14
      settings: EnsureOutportIsVirtual=on
  ActCfrPrgItem_ActCfrPrgItem_write : Outport  Port=15
      settings: EnsureOutportIsVirtual=on
  ActCfrPrgPrgGroup_ActCfrPrgPrgGroup_write : Outport  Port=16
      settings: EnsureOutportIsVirtual=on
  ActCfrPrgPrgItem_ActCfrPrgPrgItem_write : Outport  Port=17
      settings: EnsureOutportIsVirtual=on
  ActCfrPrgRef_ActCfrPrgRef_write : Outport  Port=18
      settings: EnsureOutportIsVirtual=on
  ActCfrPrgSafety_ActCfrPrgSafety_write : Outport  Port=19
      settings: EnsureOutportIsVirtual=on
  ActCfrPrgSafetyEnable_ActCfrPrgSafetyEnable_write : Outport  Port=20
      settings: EnsureOutportIsVirtual=on
  ActCfrSetAutoSpd_ActCfrSetAutoSpd_write : Outport  Port=21
      settings: EnsureOutportIsVirtual=on
  ActCfrSetFPos_ActCfrSetFPos_write : Outport  Port=22
      settings: EnsureOutportIsVirtual=on
  ActCfrSetGroup_ActCfrSetGroup_write : Outport  Port=23
      settings: EnsureOutportIsVirtual=on
  ActCfrSetItem_ActCfrSetItem_write : Outport  Port=24
      settings: EnsureOutportIsVirtual=on
  ActCfrSetLowNoise_ActCfrSetLowNoise_write : Outport  Port=25
      settings: EnsureOutportIsVirtual=on
  ActCfrSetSpd_ActCfrSetSpd_write : Outport  Port=26
      settings: EnsureOutportIsVirtual=on
  ActCfrSetStallDet_ActCfrSetStallDet_write : Outport  Port=27
      settings: EnsureOutportIsVirtual=on
  ActCfrSetStopPhs_ActCfrSetStopPhs_write : Outport  Port=28
      settings: EnsureOutportIsVirtual=on
  ActDfrIdeGroup_ActDfrIdeGroup_write : Outport  Port=29
      settings: EnsureOutportIsVirtual=on
  ActDfrIdeItem_ActDfrIdeItem_write : Outport  Port=30
      settings: EnsureOutportIsVirtual=on
  ActDfrIdeResvd_ActDfrIdeResvd_write : Outport  Port=31
      settings: EnsureOutportIsVirtual=on
  ActDfrPrgGroup_ActDfrPrgGroup_write : Outport  Port=32
      settings: EnsureOutportIsVirtual=on
  ActDfrPrgItem_ActDfrPrgItem_write : Outport  Port=33
      settings: EnsureOutportIsVirtual=on
  ActDfrPrgResvd_ActDfrPrgResvd_write : Outport  Port=34
      settings: EnsureOutportIsVirtual=on
  ActDfrStaGroup_ActDfrStaGroup_write : Outport  Port=35
      settings: EnsureOutportIsVirtual=on
  ActDfrStaItem_ActDfrStaItem_write : Outport  Port=36
      settings: EnsureOutportIsVirtual=on
  ActDfrStaResvd_ActDfrStaResvd_write : Outport  Port=37
      settings: EnsureOutportIsVirtual=on
  CcmHfaMasterReqB0Lin4_CcmHfaMasterReqB0Lin4_write : Outport  Port=38
      settings: EnsureOutportIsVirtual=on
  CcmHfaMasterReqB1Lin4_CcmHfaMasterReqB1Lin4_write : Outport  Port=39
      settings: EnsureOutportIsVirtual=on
  CcmHfaMasterReqB2Lin4_CcmHfaMasterReqB2Lin4_write : Outport  Port=40
      settings: EnsureOutportIsVirtual=on
  CcmHfaMasterReqB3Lin4_CcmHfaMasterReqB3Lin4_write : Outport  Port=41
      settings: EnsureOutportIsVirtual=on
  CcmHfaMasterReqB4Lin4_CcmHfaMasterReqB3Lin4_write : Outport  Port=42
      settings: EnsureOutportIsVirtual=on
  CcmHfaMasterReqB5Lin4_CcmHfaMasterReqB5Lin4_write : Outport  Port=43
      settings: EnsureOutportIsVirtual=on
  CcmHfaMasterReqB6Lin4_CcmHfaMasterReqB6Lin4_write : Outport  Port=44
      settings: EnsureOutportIsVirtual=on
  CcmHfaMasterReqB7Lin4_CcmHfaMasterReqB7Lin4_write : Outport  Port=45
      settings: EnsureOutportIsVirtual=on
  Lin1HfaActvReq_Lin1HfaActvReq_write : Outport  Port=46
      settings: EnsureOutportIsVirtual=on
  ReHvacMotAdr_ReHvacMotAdr_write : Outport  Port=47
      settings: EnsureOutportIsVirtual=on
  ReHvacMotBlockCmd_ReHvacMotBlockCmd_write : Outport  Port=48
      settings: EnsureOutportIsVirtual=on
  ReHvacMotClearReq_ReHvacMotClearReq_write : Outport  Port=49
      settings: EnsureOutportIsVirtual=on
  ReHvacMotCoilPwrOn_ReHvacMotCoilPwrOn_write : Outport  Port=50
      settings: EnsureOutportIsVirtual=on
  ReHvacMotDir_ReHvacMotDir_write : Outport  Port=51
      settings: EnsureOutportIsVirtual=on
  ReHvacMotMode_ReHvacMotMode_write : Outport  Port=52
      settings: EnsureOutportIsVirtual=on
  ReHvacMotPsonAvl_ReHvacMotPsonAvl_write : Outport  Port=53
      settings: EnsureOutportIsVirtual=on
  ReHvacMotSaveData_ReHvacMotSaveData_write : Outport  Port=54
      settings: EnsureOutportIsVirtual=on
  ReHvacMotSpdSet_ReHvacMotSpdSet_write : Outport  Port=55
      settings: EnsureOutportIsVirtual=on
  ReHvacMotStrtPson_ReHvacMotStrtPson_write : Outport  Port=56
      settings: EnsureOutportIsVirtual=on
  ReHvacMotTargtPson_ReHvacMotTargtPson_write : Outport  Port=57
      settings: EnsureOutportIsVirtual=on
  ReHvacMotUrgcPson_ReHvacMotUrgcPson_write : Outport  Port=58
      settings: EnsureOutportIsVirtual=on